simulink model "hyd_hyb_2_opt_cont_test_new_1_col_ab"  (Simulink 7.7)
settings: Solver=ode14x  StartTime=0  StopTime=750  FixedStep=0.01  MaxStep=.4  RelTol=1e-8
InitFcn:
  | const_3
values from neighbouring files (symbols this model uses):
  Af = 6.3    [brk_enrg.m]    (also in brk_enrg_1.m)
  BSFC_906_205 = [3.5684 4.3466 5.1668 6.0769 6.988 7.8992 8.8104 9.7336 10.8548 11.9809 13.1112 14.2571 ... (663 elements, 39x17)]    [BSFC_map_906_205.mat]
  Cd = 0.55    [brk_enrg.m]    (also in brk_enrg_1.m)
  den = 1.202    [brk_enrg.m]    (also in brk_enrg_1.m)
  fr = 0.01    [brk_enrg.m]    (also in brk_enrg_1.m)
undefined symbols (not defined in the file or in its folder): clutchRise, down_spd_2, down_spd_3, down_spd_4, down_spd_c, fuel_density, fuz_wgt, gearshift, I_wheel, Idle_spd_eng_906, M1_hydr, p_v_15, pmax_125, qv_125, Rw, sec_eng_sfc_map, T_max_906, T_min, TC_spd_ratio_max, torque_convertor, Twait, up_spd_1, up_spd_2, up_spd_3, up_spd_c, v_p_10, v_p_15, v_p_5
libraries: neural x2963, fl_lib x43, nesl_utility x30, sdl_lib x14, simulink x4, simulink_need_slupdate x1
(part 71 of 72)

== system hyd_hyb_2_opt_cont_test_new_1_col_ab/controller1/optimized controller - pm/SOC upper 0.5/SOC upper 0.6/SOC upper 0.7/SOC upper 0.8/net_pm_col_ab_89/Layer 2/LW{2,1} ==
  "ad{2,1}" : Inport  PortDimensions=23
      mask Display: e = 0.06;c1 = [120 230 180]/255;c2 = ([120 230 180]+0)/(256+128);c3 = ([120 230 180]+128)/(256+128);patch([0 1 1 0],[0 0 1 1],c1);patch([0 1 1 1-e 1-e 0+e],[0 0 1 1-e 0+e 0+e],c2);patch([0 0 1 1-e 0+e 0+e],[0 1 1 1-e 1-e 0+e],c3);plot([0 1 1 0 0],[0 0 1 1 0]);
  "IW{2,1}(1,:)'" : Constant  Value=[-2.9882769936965716;-1.9719972563463035;0.11166843136495574;-1.8506167741427433;-0.4259207812282787;-0.12336619290496181;-0.07028031333346646;1.0900319870596846;-0.74293787494109031;0.94112534866632891;0.97990959837996261;-0.74773011153265745;0.70482729889202189;-1.8043069934153724;-3.1669648937902242;0.74546489662241544;-0.8345503087286299;0.880983187410181;2.2125377542776188;0.73338545448870063;-3.0095126215890144;-0.8328861260117062;-1.4465803054412263]
      mask Display: e = 0.06;c1 = [120 150 230]/255;c2 = ([120 150 230]+0)/383;c3 = ([120 150 230]+128)/383;patch([0 1 1 0],[0 0 1 1],c1);patch([0 1 1 1-e 1-e 0+e],[0 0 1 1-e 0+e 0+e],c2);patch([0 0 1 1-e 0+e 0+e],[0 1 1 1-e 1-e 0+e],c3);plot([0 1 1 0 0],[0 0 1 1 0]);disp('weights');
  "IW{2,1}(10,:)'" : Constant  Value=[-2.5267759980618574;-1.6034673217269988;0.20101440951954702;0.7211392244393835;0.81077128059474246;-1.8633582279973888;-0.65573965450509342;-0.23330320545973349;1.5366465477495479;1.5284005273710839;2.6040230618015943;-1.3460656488847356;-0.94799934668319175;-2.6097819378086111;-1.4360269518974962;0.12988154533478583;-0.0511454543380363;-1.3227729890974305;-2.3814271236997588;-0.13506646628624136;-3.3308404627428687;0.93457748143530084;1.2371184186316071]
      mask Display: e = 0.06;c1 = [120 150 230]/255;c2 = ([120 150 230]+0)/383;c3 = ([120 150 230]+128)/383;patch([0 1 1 0],[0 0 1 1],c1);patch([0 1 1 1-e 1-e 0+e],[0 0 1 1-e 0+e 0+e],c2);patch([0 0 1 1-e 0+e 0+e],[0 1 1 1-e 1-e 0+e],c3);plot([0 1 1 0 0],[0 0 1 1 0]);disp('weights');
  "IW{2,1}(11,:)'" : Constant  Value=[0.56213571851403787;-2.1440908402256706;-1.4054644214019001;0.41050464577895862;1.808011142764296;-0.02452565250699737;2.8654415674512292;0.44421705575756476;1.1347817902517405;1.4638698996107873;-1.7293602502974175;-0.73088620281693695;-2.0533735940908495;-1.1833814533359843;1.4587537931904402;-2.2945169599365141;0.31547399387798558;-0.42021555273887057;2.9178367416561053;-1.5357044275683207;-2.9446389712357761;1.7138426100138346;-0.78419393822899208]
      mask Display: e = 0.06;c1 = [120 150 230]/255;c2 = ([120 150 230]+0)/383;c3 = ([120 150 230]+128)/383;patch([0 1 1 0],[0 0 1 1],c1);patch([0 1 1 1-e 1-e 0+e],[0 0 1 1-e 0+e 0+e],c2);patch([0 0 1 1-e 0+e 0+e],[0 1 1 1-e 1-e 0+e],c3);plot([0 1 1 0 0],[0 0 1 1 0]);disp('weights');
  "IW{2,1}(12,:)'" : Constant  Value=[3.8459419827357042;-1.565125807364834;3.4079270670841892;-1.0965473812354933;1.3990795355953667;-5.7121611072747234;1.9484948998615057;-1.237959578657958;2.0546576630538764;-2.4400567797092481;-1.8426707682602037;-6.3090683358451667;-2.1320027636892793;-0.86702237488811629;-5.019581583941255;3.0766110594128659;2.4065899476778179;1.0034301645890289;-2.0969532099165149;0.36245753703309475;1.7568154487975161;1.4537550233329515;1.6195184529022357]
      mask Display: e = 0.06;c1 = [120 150 230]/255;c2 = ([120 150 230]+0)/383;c3 = ([120 150 230]+128)/383;patch([0 1 1 0],[0 0 1 1],c1);patch([0 1 1 1-e 1-e 0+e],[0 0 1 1-e 0+e 0+e],c2);patch([0 0 1 1-e 0+e 0+e],[0 1 1 1-e 1-e 0+e],c3);plot([0 1 1 0 0],[0 0 1 1 0]);disp('weights');
  "IW{2,1}(13,:)'" : Constant  Value=[0.90119872575119098;-1.8542891696752677;-1.428230614678619;-4.8923539470384982;1.3220870585176394;-4.9546469794609598;2.1416339015688584;1.9768800725972122;1.8641621350781945;-0.96723635955835063;-2.9107571124394878;-3.1557183353168092;0.14299914768574457;-2.6813336610932219;-0.36827479719921419;3.2103702298302816;6.0666508332783859;1.1007272475974077;7.9887459119138891;-0.9678195415676949;-0.72401992864216846;-1.2029094694888771;-2.1118498890487758]
      mask Display: e = 0.06;c1 = [120 150 230]/255;c2 = ([120 150 230]+0)/383;c3 = ([120 150 230]+128)/383;patch([0 1 1 0],[0 0 1 1],c1);patch([0 1 1 1-e 1-e 0+e],[0 0 1 1-e 0+e 0+e],c2);patch([0 0 1 1-e 0+e 0+e],[0 1 1 1-e 1-e 0+e],c3);plot([0 1 1 0 0],[0 0 1 1 0]);disp('weights');
  "IW{2,1}(14,:)'" : Constant  Value=[0.28145866221881538;-0.23131432154620815;1.9025758923533957;1.5443354004516294;0.14307592475137126;-0.084865785845065267;-1.5677774808597273;-2.1664113374800582;-1.8570926782130321;0.40066182733363714;1.1053874895003304;0.10713803752989356;-0.95713301681979879;-0.036361364085088804;1.7599026454816242;-0.64973268342196255;-2.6426963786636399;1.7800397318573569;-1.9170588699450515;-1.0026102656894991;2.2382783368930661;0.98103583646760129;1.4445906752096962]
      mask Display: e = 0.06;c1 = [120 150 230]/255;c2 = ([120 150 230]+0)/383;c3 = ([120 150 230]+128)/383;patch([0 1 1 0],[0 0 1 1],c1);patch([0 1 1 1-e 1-e 0+e],[0 0 1 1-e 0+e 0+e],c2);patch([0 0 1 1-e 0+e 0+e],[0 1 1 1-e 1-e 0+e],c3);plot([0 1 1 0 0],[0 0 1 1 0]);disp('weights');
  "IW{2,1}(15,:)'" : Constant  Value=[0.83347172432809657;-0.57618710618521174;-1.0178470438223421;1.3806523177250209;-2.8643786217684699;-1.6036473779305813;-1.9283933613918303;1.5147059324272705;0.026543732605154406;-0.16765487558918132;0.86867148514494619;2.0932333855539724;0.76339298762694785;-0.18865011542113022;4.3703690465067888;2.3970242795282477;-0.73309161401245959;2.4341436793201514;1.4262652838451537;-1.8818476199236884;0.15749440453226543;1.3712136727373807;1.2376588370362307]
      mask Display: e = 0.06;c1 = [120 150 230]/255;c2 = ([120 150 230]+0)/383;c3 = ([120 150 230]+128)/383;patch([0 1 1 0],[0 0 1 1],c1);patch([0 1 1 1-e 1-e 0+e],[0 0 1 1-e 0+e 0+e],c2);patch([0 0 1 1-e 0+e 0+e],[0 1 1 1-e 1-e 0+e],c3);plot([0 1 1 0 0],[0 0 1 1 0]);disp('weights');
  "IW{2,1}(16,:)'" : Constant  Value=[2.455738623909332;0.32197591610739157;1.0355188451621173;2.7664643176780044;2.1940148039182512;1.122963087059617;0.093132262147403583;2.4965637902632909;1.3589750632743591;1.2266850685195245;-0.024938627361850919;1.0796646796187448;-1.1729484301265563;-0.033926811235205669;-0.90620793673587785;-0.85908930515572335;0.99401587915799716;2.1722793936799341;-1.3589625341661014;-0.55750444061892723;0.019808687609242023;2.0392530333827663;3.1330547954635732]
      mask Display: e = 0.06;c1 = [120 150 230]/255;c2 = ([120 150 230]+0)/383;c3 = ([120 150 230]+128)/383;patch([0 1 1 0],[0 0 1 1],c1);patch([0 1 1 1-e 1-e 0+e],[0 0 1 1-e 0+e 0+e],c2);patch([0 0 1 1-e 0+e 0+e],[0 1 1 1-e 1-e 0+e],c3);plot([0 1 1 0 0],[0 0 1 1 0]);disp('weights');
  "IW{2,1}(17,:)'" : Constant  Value=[0.7348029250667053;-0.071666647846748199;0.71432343624593486;2.0357169989376671;-2.5985322564875157;0.71879520391505314;1.8146057566181226;2.8802861177728767;1.4605664883182157;-0.53464257579970287;1.4501419041584109;-3.233376223329349;-0.15300066025637604;-1.3263849397077898;-3.5107341578368256;-0.6723631781467867;0.27719843687473589;1.5249190470838518;1.4097765069456738;0.64277328966585623;1.1317449011083349;-1.4447262949902151;-0.34463527790460208]
      mask Display: e = 0.06;c1 = [120 150 230]/255;c2 = ([120 150 230]+0)/383;c3 = ([120 150 230]+128)/383;patch([0 1 1 0],[0 0 1 1],c1);patch([0 1 1 1-e 1-e 0+e],[0 0 1 1-e 0+e 0+e],c2);patch([0 0 1 1-e 0+e 0+e],[0 1 1 1-e 1-e 0+e],c3);plot([0 1 1 0 0],[0 0 1 1 0]);disp('weights');
  "IW{2,1}(18,:)'" : Constant  Value=[1.7698345444801302;-1.0300163057514935;0.27846112378675092;-0.25700476483626544;-1.6102663001616404;2.2563152183804753;2.2672924258125304;-0.25730415259313966;-2.066325507522027;-2.0154339046862555;1.4218884853478149;0.78550746224611112;-0.69728374295602424;0.20907495933017475;1.189604158118551;0.90528968362595086;-0.16531496791075728;1.1453903742274976;1.129583640768564;-1.7943207500837921;-1.7718486569305396;-1.1929266226045185;-1.6940717464707511]
      mask Display: e = 0.06;c1 = [120 150 230]/255;c2 = ([120 150 230]+0)/383;c3 = ([120 150 230]+128)/383;patch([0 1 1 0],[0 0 1 1],c1);patch([0 1 1 1-e 1-e 0+e],[0 0 1 1-e 0+e 0+e],c2);patch([0 0 1 1-e 0+e 0+e],[0 1 1 1-e 1-e 0+e],c3);plot([0 1 1 0 0],[0 0 1 1 0]);disp('weights');
  "IW{2,1}(19,:)'" : Constant  Value=[1.1768994688211867;0.37058476452098071;0.6484760889556116;-0.55324017865954178;-1.5253366454680874;1.4571386429182389;-1.3410939502623833;2.180224193479821;1.4262832205082852;-1.4484595961867182;1.0754137600264355;2.1163146313424663;1.8537505703509642;-1.4929584181653344;1.3803371781158784;1.3828723567313919;0.57370481354639014;1.1021719879131213;-1.5128504211335914;2.0670668425140435;-0.21901192525761012;2.4164076765144453;1.7835064001293242]
      mask Display: e = 0.06;c1 = [120 150 230]/255;c2 = ([120 150 230]+0)/383;c3 = ([120 150 230]+128)/383;patch([0 1 1 0],[0 0 1 1],c1);patch([0 1 1 1-e 1-e 0+e],[0 0 1 1-e 0+e 0+e],c2);patch([0 0 1 1-e 0+e 0+e],[0 1 1 1-e 1-e 0+e],c3);plot([0 1 1 0 0],[0 0 1 1 0]);disp('weights');
  "IW{2,1}(2,:)'" : Constant  Value=[2.4804331520952396;1.6056595948886279;-2.5886708230182571;2.9595162565766402;0.67543849624771257;-0.23620294500460123;-0.84011376145360583;3.6762798139747659;3.322675493556555;-0.36354469847575765;-0.00067879346676537333;3.6457383570356812;0.84397444659638798;-2.2805650729513429;1.2396872744380787;-1.8679582469817573;-2.2552605065294067;0.22312163569475774;-0.93963127596051255;-1.8001214318249512;-0.37517972743200834;-3.9890511709290348;0.9040001462483841]
      mask Display: e = 0.06;c1 = [120 150 230]/255;c2 = ([120 150 230]+0)/383;c3 = ([120 150 230]+128)/383;patch([0 1 1 0],[0 0 1 1],c1);patch([0 1 1 1-e 1-e 0+e],[0 0 1 1-e 0+e 0+e],c2);patch([0 0 1 1-e 0+e 0+e],[0 1 1 1-e 1-e 0+e],c3);plot([0 1 1 0 0],[0 0 1 1 0]);disp('weights');
  "IW{2,1}(20,:)'" : Constant  Value=[-0.73819203710871928;2.2387092772460688;-0.49819903087560513;-3.0560697684227418;-0.50692692141884799;1.9036949466408606;1.4491557711689345;0.5255026104516699;3.0436160139161368;-0.101105209113682;0.11276983637476454;0.52357844060741066;-1.4875859542548575;-2.2026804780460596;-2.7658597266904579;1.7530133614571799;-2.5375945963987525;-0.78530748294641428;0.98676034054523787;-0.14915769161493342;-2.1036903742828286;1.2225391735640321;0.24643636332414248]
      mask Display: e = 0.06;c1 = [120 150 230]/255;c2 = ([120 150 230]+0)/383;c3 = ([120 150 230]+128)/383;patch([0 1 1 0],[0 0 1 1],c1);patch([0 1 1 1-e 1-e 0+e],[0 0 1 1-e 0+e 0+e],c2);patch([0 0 1 1-e 0+e 0+e],[0 1 1 1-e 1-e 0+e],c3);plot([0 1 1 0 0],[0 0 1 1 0]);disp('weights');
  "IW{2,1}(3,:)'" : Constant  Value=[0.1801589565316721;0.96410588767084038;1.8216564108827107;0.85742882997216907;-3.0475906864883715;1.9483322348222001;-2.5841168114746429;-0.93877103497062875;-2.2089179737851268;-0.85447414746448624;-0.84352636440367101;1.1242375792814352;2.4486709799180386;-0.50958013100151833;1.607541790469651;1.4114199002024013;-0.43352761297502823;0.092110772228179399;0.31330273479979515;0.98208771750988333;-0.7868855347589665;-0.3406459635627872;-0.36962905086721592]
      mask Display: e = 0.06;c1 = [120 150 230]/255;c2 = ([120 150 230]+0)/383;c3 = ([120 150 230]+128)/383;patch([0 1 1 0],[0 0 1 1],c1);patch([0 1 1 1-e 1-e 0+e],[0 0 1 1-e 0+e 0+e],c2);patch([0 0 1 1-e 0+e 0+e],[0 1 1 1-e 1-e 0+e],c3);plot([0 1 1 0 0],[0 0 1 1 0]);disp('weights');
  "IW{2,1}(4,:)'" : Constant  Value=[0.81160839739885238;-1.6491678232454614;1.4616109513244708;1.6568093131131418;0.61559920685367664;-3.5117765792663862;-0.049871837583293027;-0.22976309509996312;-1.2478798860521347;0.63745863833413052;-2.7487281961560459;0.19048877044771362;1.7053203540894144;0.1501837187035516;0.28506723844169973;2.91527668363095;1.6294298626988437;3.2832112436619658;1.4170196406654252;-0.42361194349768883;-1.2985444501418058;1.9621712886563762;-0.83664724440521332]
      mask Display: e = 0.06;c1 = [120 150 230]/255;c2 = ([120 150 230]+0)/383;c3 = ([120 150 230]+128)/383;patch([0 1 1 0],[0 0 1 1],c1);patch([0 1 1 1-e 1-e 0+e],[0 0 1 1-e 0+e 0+e],c2);patch([0 0 1 1-e 0+e 0+e],[0 1 1 1-e 1-e 0+e],c3);plot([0 1 1 0 0],[0 0 1 1 0]);disp('weights');
  "IW{2,1}(5,:)'" : Constant  Value=[-1.6656730494057617;2.101728722417608;-3.6523490993384158;-1.6844514245319118;-0.056609872614464395;3.3773938308783924;-2.857484238042157;-1.4177669399067419;5.1000148722484857;1.5439910732434561;5.3869760273352671;-1.7704758107986673;-1.4928471557945966;0.82650174710805047;3.0138830208286378;0.9729136287633412;2.1842431722958189;-1.4286637608978807;-0.30605043066803139;-0.89754804799567278;-4.7419480627682518;-2.3350616623714817;-0.33544987987522185]
      mask Display: e = 0.06;c1 = [120 150 230]/255;c2 = ([120 150 230]+0)/383;c3 = ([120 150 230]+128)/383;patch([0 1 1 0],[0 0 1 1],c1);patch([0 1 1 1-e 1-e 0+e],[0 0 1 1-e 0+e 0+e],c2);patch([0 0 1 1-e 0+e 0+e],[0 1 1 1-e 1-e 0+e],c3);plot([0 1 1 0 0],[0 0 1 1 0]);disp('weights');
  "IW{2,1}(6,:)'" : Constant  Value=[-0.45003034679056431;-0.1899145417884309;-0.19151051949353523;1.9502018648853188;2.7306077460157212;2.2723378112026236;-0.056072026487968191;-2.7504377261417527;0.066000132504918874;2.2575034025883673;2.386296652450429;3.7211409127635879;-0.30996737220687831;0.52391677091123834;-0.20677895093508489;0.87734988084337906;1.8596340337690462;1.7013406309074697;0.81425212854243245;-0.10627069296864451;0.68400199087999769;-0.40402188477552392;1.6655740508024803]
      mask Display: e = 0.06;c1 = [120 150 230]/255;c2 = ([120 150 230]+0)/383;c3 = ([120 150 230]+128)/383;patch([0 1 1 0],[0 0 1 1],c1);patch([0 1 1 1-e 1-e 0+e],[0 0 1 1-e 0+e 0+e],c2);patch([0 0 1 1-e 0+e 0+e],[0 1 1 1-e 1-e 0+e],c3);plot([0 1 1 0 0],[0 0 1 1 0]);disp('weights');
  "IW{2,1}(7,:)'" : Constant  Value=[0.48117505017019191;1.7178171071332622;0.44379227016524803;-0.70741836271827774;-2.480605206861425;-2.8392470801005789;0.32747110326963391;-2.1401091008259625;2.5442448569038048;0.76679025461761541;-1.1746928689428493;0.2378798259979685;3.1835674584948555;2.1954814615985181;-1.0795722988864846;0.74807555750643329;-2.2842821203049972;-1.5189415095071468;1.1878205552701424;-2.0887434766647179;1.1595033211582439;-0.54579012646707648;-1.2121777209687368]
      mask Display: e = 0.06;c1 = [120 150 230]/255;c2 = ([120 150 230]+0)/383;c3 = ([120 150 230]+128)/383;patch([0 1 1 0],[0 0 1 1],c1);patch([0 1 1 1-e 1-e 0+e],[0 0 1 1-e 0+e 0+e],c2);patch([0 0 1 1-e 0+e 0+e],[0 1 1 1-e 1-e 0+e],c3);plot([0 1 1 0 0],[0 0 1 1 0]);disp('weights');
  "IW{2,1}(8,:)'" : Constant  Value=[-0.68364040657043479;-1.0284745138722959;-3.5306549663944069;-1.626378664916019;-0.48573042383266946;-4.5287585211666066;-1.8333318293584757;-0.27468348716286939;2.809119243529631;0.88564236032648969;-3.0143734224782075;6.0754694513432943;-0.75177367132108264;4.9483227897931261;1.0670043249700369;0.17975691676975469;-0.4336332217245315;-0.76603772244000112;0.86744531060230323;-0.8558953805914552;-2.4286291694065882;1.6594682683698436;0.23754536997907844]
      mask Display: e = 0.06;c1 = [120 150 230]/255;c2 = ([120 150 230]+0)/383;c3 = ([120 150 230]+128)/383;patch([0 1 1 0],[0 0 1 1],c1);patch([0 1 1 1-e 1-e 0+e],[0 0 1 1-e 0+e 0+e],c2);patch([0 0 1 1-e 0+e 0+e],[0 1 1 1-e 1-e 0+e],c3);plot([0 1 1 0 0],[0 0 1 1 0]);disp('weights');
  "IW{2,1}(9,:)'" : Constant  Value=[1.2323596528686418;-0.8834794834224613;3.1793058422467948;0.73885962810267047;-0.23179395997337127;0.80805621708291953;-0.063299249154589121;1.2782356953410301;4.7144332993068394;-0.095642216754081799;0.92954818661376781;-3.158425303374;-0.49937467719287176;-1.7643472538310863;2.527211503081467;2.1502863478250522;0.79045868295966848;-0.6991837381809215;0.65964383753608458;-2.0650941741915041;-0.53260986575500935;-1.085565102984887;-1.8763997392285039]
      mask Display: e = 0.06;c1 = [120 150 230]/255;c2 = ([120 150 230]+0)/383;c3 = ([120 150 230]+128)/383;patch([0 1 1 0],[0 0 1 1],c1);patch([0 1 1 1-e 1-e 0+e],[0 0 1 1-e 0+e 0+e],c2);patch([0 0 1 1-e 0+e 0+e],[0 1 1 1-e 1-e 0+e],c3);plot([0 1 1 0 0],[0 0 1 1 0]);disp('weights');
  Mux : Mux  Inputs=20
      mask Display: e = 0.06;c1 = [120 150 230]/255;c2 = ([120 150 230]+0)/383;c3 = ([120 150 230]+128)/383;patch([0 1 1 0],[0 0 1 1],c1);patch([0 1 1 1-e 1-e 0+e],[0 0 1 1-e 0+e 0+e],c2);patch([0 0 1 1-e 0+e 0+e],[0 1 1 1-e 1-e 0+e],c3);plot([0 1 1 0 0],[0 0 1 1 0]);disp('Mux');
  dotprod{1..20} (20 alike) : neural/Weight Functions/dotprod  SystemSampleTime=-1
  "lz{2,1}" : Outport
      mask Display: e = 0.06;c1 = [120 230 180]/255;c2 = ([120 230 180]+0)/(256+128);c3 = ([120 230 180]+128)/(256+128);patch([0 1 1 0],[0 0 1 1],c1);patch([0 1 1 1-e 1-e 0+e],[0 0 1 1-e 0+e 0+e],c2);patch([0 0 1 1-e 0+e 0+e],[0 1 1 1-e 1-e 0+e],c3);plot([0 1 1 0 0],[0 0 1 1 0]);
  wires:
  "IW{2,1}(1,:)'"/1 -> dotprod1/1
  "ad{2,1}"/1 -> dotprod1/2, dotprod2/2, dotprod3/2, dotprod4/2, dotprod5/2, dotprod6/2, dotprod7/2, dotprod8/2, dotprod9/2, dotprod10/2, dotprod11/2, dotprod12/2, dotprod13/2, dotprod14/2, dotprod15/2, dotprod16/2, dotprod17/2, dotprod18/2, dotprod19/2, dotprod20/2
  dotprod1/1 -> Mux/1
  "IW{2,1}(2,:)'"/1 -> dotprod2/1
  dotprod2/1 -> Mux/2
  "IW{2,1}(3,:)'"/1 -> dotprod3/1
  dotprod3/1 -> Mux/3
  "IW{2,1}(4,:)'"/1 -> dotprod4/1
  dotprod4/1 -> Mux/4
  "IW{2,1}(5,:)'"/1 -> dotprod5/1
  dotprod5/1 -> Mux/5
  "IW{2,1}(6,:)'"/1 -> dotprod6/1
  dotprod6/1 -> Mux/6
  "IW{2,1}(7,:)'"/1 -> dotprod7/1
  dotprod7/1 -> Mux/7
  "IW{2,1}(8,:)'"/1 -> dotprod8/1
  dotprod8/1 -> Mux/8
  "IW{2,1}(9,:)'"/1 -> dotprod9/1
  dotprod9/1 -> Mux/9
  "IW{2,1}(10,:)'"/1 -> dotprod10/1
  dotprod10/1 -> Mux/10
  "IW{2,1}(11,:)'"/1 -> dotprod11/1
  dotprod11/1 -> Mux/11
  "IW{2,1}(12,:)'"/1 -> dotprod12/1
  dotprod12/1 -> Mux/12
  "IW{2,1}(13,:)'"/1 -> dotprod13/1
  dotprod13/1 -> Mux/13
  "IW{2,1}(14,:)'"/1 -> dotprod14/1
  dotprod14/1 -> Mux/14
  "IW{2,1}(15,:)'"/1 -> dotprod15/1
  dotprod15/1 -> Mux/15
  "IW{2,1}(16,:)'"/1 -> dotprod16/1
  dotprod16/1 -> Mux/16
  "IW{2,1}(17,:)'"/1 -> dotprod17/1
  dotprod17/1 -> Mux/17
  "IW{2,1}(18,:)'"/1 -> dotprod18/1
  dotprod18/1 -> Mux/18
  "IW{2,1}(19,:)'"/1 -> dotprod19/1
  dotprod19/1 -> Mux/19
  "IW{2,1}(20,:)'"/1 -> dotprod20/1
  dotprod20/1 -> Mux/20
  Mux/1 -> "lz{2,1}"/1

== system hyd_hyb_2_opt_cont_test_new_1_col_ab/controller1/optimized controller - pm/SOC upper 0.5/SOC upper 0.6/SOC upper 0.7/SOC upper 0.8/net_pm_col_ab_89/Layer 3/Delays 1 ==
  "p{3}" : Inport  PortDimensions=20
      mask Display: e = 0.06;c1 = [120 230 180]/255;c2 = ([120 230 180]+0)/(256+128);c3 = ([120 230 180]+128)/(256+128);patch([0 1 1 0],[0 0 1 1],c1);patch([0 1 1 1-e 1-e 0+e],[0 0 1 1-e 0+e 0+e],c2);patch([0 0 1 1-e 0+e 0+e],[0 1 1 1-e 1-e 0+e],c3);plot([0 1 1 0 0],[0 0 1 1 0]);
  mux : Mux  Inputs=1
      mask Display: e = 0.06;c1 = [120 150 230]/255;c2 = ([120 150 230]+0)/383;c3 = ([120 150 230]+128)/383;patch([0 1 1 0],[0 0 1 1],c1);patch([0 1 1 1-e 1-e 0+e],[0 0 1 1-e 0+e 0+e],c2);patch([0 0 1 1-e 0+e 0+e],[0 1 1 1-e 1-e 0+e],c3);plot([0 1 1 0 0],[0 0 1 1 0]);disp('Mux')
  "pd{3,2}" : Outport
      mask Display: e = 0.06;c1 = [120 230 180]/255;c2 = ([120 230 180]+0)/(256+128);c3 = ([120 230 180]+128)/(256+128);patch([0 1 1 0],[0 0 1 1],c1);patch([0 1 1 1-e 1-e 0+e],[0 0 1 1-e 0+e 0+e],c2);patch([0 0 1 1-e 0+e 0+e],[0 1 1 1-e 1-e 0+e],c3);plot([0 1 1 0 0],[0 0 1 1 0]);
  wires:
  "p{3}"/1 -> mux/1
  mux/1 -> "pd{3,2}"/1

== system hyd_hyb_2_opt_cont_test_new_1_col_ab/controller1/optimized controller - pm/SOC upper 0.5/SOC upper 0.6/SOC upper 0.7/SOC upper 0.8/net_pm_col_ab_89/Layer 3/LW{3,2} ==
  "ad{3,2}" : Inport  PortDimensions=20
      mask Display: e = 0.06;c1 = [120 230 180]/255;c2 = ([120 230 180]+0)/(256+128);c3 = ([120 230 180]+128)/(256+128);patch([0 1 1 0],[0 0 1 1],c1);patch([0 1 1 1-e 1-e 0+e],[0 0 1 1-e 0+e 0+e],c2);patch([0 0 1 1-e 0+e 0+e],[0 1 1 1-e 1-e 0+e],c3);plot([0 1 1 0 0],[0 0 1 1 0]);
  "IW{3,2}(1,:)'" : Constant  Value=[-1.3061056718343567;-3.7020477296756877;-0.81135149977132004;-5.0740443485098403;-3.8030524254892999;-1.7062817057967512;-0.14165213560801915;4.5794145827239046;3.6993044531061288;1.7309251819330165;-0.2060736594675476;-4.5644001795897902;5.1428384754283893;2.8978531504094502;-1.7934953746916185;0.70501448565099145;3.7557650229782267;0.94020981359969125;-0.48154557452183244;-2.0482795826800908]
      mask Display: e = 0.06;c1 = [120 150 230]/255;c2 = ([120 150 230]+0)/383;c3 = ([120 150 230]+128)/383;patch([0 1 1 0],[0 0 1 1],c1);patch([0 1 1 1-e 1-e 0+e],[0 0 1 1-e 0+e 0+e],c2);patch([0 0 1 1-e 0+e 0+e],[0 1 1 1-e 1-e 0+e],c3);plot([0 1 1 0 0],[0 0 1 1 0]);disp('weights');
  Mux : Mux  Inputs=1
      mask Display: e = 0.06;c1 = [120 150 230]/255;c2 = ([120 150 230]+0)/383;c3 = ([120 150 230]+128)/383;patch([0 1 1 0],[0 0 1 1],c1);patch([0 1 1 1-e 1-e 0+e],[0 0 1 1-e 0+e 0+e],c2);patch([0 0 1 1-e 0+e 0+e],[0 1 1 1-e 1-e 0+e],c3);plot([0 1 1 0 0],[0 0 1 1 0]);disp('Mux');
  dotprod1 : neural/Weight Functions/dotprod  SystemSampleTime=-1
  "lz{3,2}" : Outport
      mask Display: e = 0.06;c1 = [120 230 180]/255;c2 = ([120 230 180]+0)/(256+128);c3 = ([120 230 180]+128)/(256+128);patch([0 1 1 0],[0 0 1 1],c1);patch([0 1 1 1-e 1-e 0+e],[0 0 1 1-e 0+e 0+e],c2);patch([0 0 1 1-e 0+e 0+e],[0 1 1 1-e 1-e 0+e],c3);plot([0 1 1 0 0],[0 0 1 1 0]);
  wires:
  "IW{3,2}(1,:)'"/1 -> dotprod1/1
  "ad{3,2}"/1 -> dotprod1/2
  dotprod1/1 -> Mux/1
  Mux/1 -> "lz{3,2}"/1

== system hyd_hyb_2_opt_cont_test_new_1_col_ab/controller1/optimized controller - pm/SOC upper 0.5/SOC upper 0.6/SOC upper 0.7/SOC upper 0.8/net_pm_col_ab_89_a/Layer 1/Delays 1 ==
  "p{1}" : Inport  PortDimensions=2
      mask Display: e = 0.06;c1 = [120 230 180]/255;c2 = ([120 230 180]+0)/(256+128);c3 = ([120 230 180]+128)/(256+128);patch([0 1 1 0],[0 0 1 1],c1);patch([0 1 1 1-e 1-e 0+e],[0 0 1 1-e 0+e 0+e],c2);patch([0 0 1 1-e 0+e 0+e],[0 1 1 1-e 1-e 0+e],c3);plot([0 1 1 0 0],[0 0 1 1 0]);
  mux : Mux  Inputs=1
      mask Display: e = 0.06;c1 = [120 150 230]/255;c2 = ([120 150 230]+0)/383;c3 = ([120 150 230]+128)/383;patch([0 1 1 0],[0 0 1 1],c1);patch([0 1 1 1-e 1-e 0+e],[0 0 1 1-e 0+e 0+e],c2);patch([0 0 1 1-e 0+e 0+e],[0 1 1 1-e 1-e 0+e],c3);plot([0 1 1 0 0],[0 0 1 1 0]);disp('Mux')
  "pd{1,1}" : Outport
      mask Display: e = 0.06;c1 = [120 230 180]/255;c2 = ([120 230 180]+0)/(256+128);c3 = ([120 230 180]+128)/(256+128);patch([0 1 1 0],[0 0 1 1],c1);patch([0 1 1 1-e 1-e 0+e],[0 0 1 1-e 0+e 0+e],c2);patch([0 0 1 1-e 0+e 0+e],[0 1 1 1-e 1-e 0+e],c3);plot([0 1 1 0 0],[0 0 1 1 0]);
  wires:
  "p{1}"/1 -> mux/1
  mux/1 -> "pd{1,1}"/1
